simulink model "controller_observer_AllAgents_v5"  (Simulink 8.4)
settings: Solver=ode1  StartTime=0.0  StopTime=inf  FixedStep=60*1  RelTol=1e-3
values from neighbouring files (symbols this model uses):
  Cpw = 4180    [readepJSONout.m]
  m_max = 3    [DesignParameters.m]
  mc_MAX = 2    [DesignParameters.m]
  Tamb_additive_Sensor_fault_time = 60*60*24*(Tamb_day_ofthe_add_fault + Tamb_time_ofthe_add_fault)    (= 129600)    [faults_setting.m]
  Tamb_additive_Sensor_fault_value = 10    [faults_setting.m]
  Tamb_mult_Sensor_fault_time = 60*60*24*(Tamb_day_ofthe_mult_fault + Tamb_time_ofthe_mult_fault)    (= 129600)    [faults_setting.m]
  Tamb_mult_Sensor_fault_value = 1.1    [faults_setting.m]
  Ts = 3600/dat.Timestep.Timestep_0x20_1.number_of_timesteps_per_hour    [readepJSONout.m]
  Tst_C_additive_Sensor_fault_time = 60*60*24*(Tst_C_day_ofthe_add_fault + Tst_C_time_ofthe_add_fault)    (= 129600)    [faults_setting.m]
  Tst_C_additive_Sensor_fault_value = 10    [faults_setting.m]
  Tst_C_mult_Sensor_fault_time = 60*60*24*(Tst_C_day_ofthe_mult_fault + Tst_C_time_ofthe_mult_fault)    (= 129600)    [faults_setting.m]
  Tst_C_mult_Sensor_fault_value = 1.1    [faults_setting.m]
  Tst_H_additive_Sensor_fault_time = 60*60*24*(Tst_H_day_ofthe_add_fault + Tst_H_time_ofthe_add_fault)    (= 129600)    [faults_setting.m]
  Tst_H_additive_Sensor_fault_value = 10    [faults_setting.m]
  Tst_H_mult_Sensor_fault_time = 60*60*24*(Tst_H_day_ofthe_mult_fault + Tst_H_time_ofthe_mult_fault)    (= 129600)    [faults_setting.m]
  Tst_H_mult_Sensor_fault_value = 1.1    [faults_setting.m]
undefined symbols (not defined in the file or in its folder): building, COP, Ehp_max, fsa_E, fsa_ET, fsa_N, fsa_NT, fsa_W, fsa_WT, h_E, h_ET, h_N, h_NT, h_W, h_WT, K_NtoNT, K_NTtoN, Ke_E, Ke_ET, Ke_N, Ke_NT, Ke_WT, Km_E, Km_ET, Km_N, Km_NT, Km_W, Km_WT, Kout_N, Kout_NT, Kout_W, Kout_WT, Kst, Ksta, Kstcij, KstRef, Kw_E, Kw_ET, Kw_N, Kw_NT, Kw_W, Kw_WT
libraries: simulink x61
(part 28 of 36)

== system controller_observer_AllAgents_v5/Controller/CorridorPod1_Agent/Threshold_zone ==
  3 : Constant  SampleTime=Ts
  4 : Constant  Value=[ones(numel(building.zone(10).neighbour),1)]  SampleTime=Ts
  Add2 : Sum  Inputs=+-
  Add3 : Sum  Inputs=+-
  Divide : Product  Inputs=*/
  Dot Product1 : DotProduct  SampleTime=Ts  InputSameDT=on  OutDataTypeStr=Inherit: Inherit via internal rule
  Fcn2 : Fcn  Expr=u(2)^(u(1))  SampleTime=Ts
  Gain : Gain  Gain=building.zone(10).e_bar  SampleTime=Ts
  If Action Subsystem2 : Subsystem  TreatAsAtomicUnit=on
  If Action Subsystem3 : Subsystem  TreatAsAtomicUnit=on
  If1 : If  IfExpression=u1 == 0  SampleTime=Ts
  Merge : Merge  InitialOutput=[building.zone(4).e_bar+building.zone(4).n_bar]
  Mux2 : Mux  Inputs=2
  Mux3 : Mux  Inputs=[1 numel(building.zone(10).neighbour) 1 1]
  Product1 : Product  SampleTime=Ts
  Product5 : Product  SampleTime=Ts
  Sum3 : Sum  Inputs=|++  SampleTime=Ts
  Sum4 : Sum  Inputs=+++  SampleTime=Ts
  ZoneGainsThreshold1 : Constant  Value=[building.zone(10).B_z; transpose(building.zone(10).B_z*building.zone(10).n_nei.*building.zone(10).Kij); building.zone(10).B_z*building.zone(10).L; building.zone(10).B_z*building.zone(10).Qbar]  SampleTime=Ts  FramePeriod=Ts
  a mat : ToFile  Filename=C:\Users\papad\Dropbox\Complete_building_simulator_Observers\Results_fz4_sensor\aH_CorridorPod1.mat  SaveFormat=Timeseries  SampleTime=Ts
  a1 : Constant  Value=building.zone(10).a  SampleTime=Ts
  ae1 : Constant  Value=building.zone(10).a*building.zone(10).e_bar  SampleTime=Ts
  ae_sum1 : ToFile  Filename=C:\Users\papad\Dropbox\Complete_building_simulator_Observers\Results_fz4_sensor\aeH_sum_CorridorPod1.mat  SaveFormat=Timeseries  SampleTime=Ts
  ae_z2 : ToFile  Filename=C:\Users\papad\Dropbox\Complete_building_simulator_Observers\Results_fz4_sensor\aeH_CorridorPod1.mat  SaveFormat=Timeseries  SampleTime=Ts
  ae_z3 : ToFile  Filename=C:\Users\papad\Dropbox\Complete_building_simulator_Observers\Results_fz4_sensor\kH_CorridorPod1.mat  SaveFormat=Timeseries  SampleTime=Ts
  counter : Subsystem
  n_bar1 : Constant  Value=building.zone(10).n_bar  SampleTime=Ts
  n_bar2 : Constant  Value=building.zone(4).n_bar  SampleTime=Ts
  x1 : ToFile  Filename=C:\Users\papad\Dropbox\Complete_building_simulator_Observers\Results_fz4_sensor\xH_CorridorPod1.mat  SaveFormat=Timeseries  SampleTime=Ts
  Out1 : Outport
  wires:
  Mux3/1 -> Dot Product1/1
  ZoneGainsThreshold1/1 -> Dot Product1/2
  n_bar1/1 -> Mux3/1
  4/1 -> Mux3/2
  3/1 -> Mux3/4
  If1/1 -> If Action Subsystem2/Action
  If1/2 -> If Action Subsystem3/Action
  If Action Subsystem2/1(Out1) -> Merge/1  "k=0"
  If Action Subsystem3/1(Out1) -> Merge/2  "k>0"
  Sum3/1 -> If Action Subsystem2/1(In1)
  Sum4/1 -> If Action Subsystem3/1(In1)
  Gain/1 -> Sum4/1, ae_z2/1
  Fcn2/1 -> Add2/2, Gain/1  "a^k"
  Dot Product1/1 -> Product5/2, x1/1  "x"
  Product5/1 -> Sum4/3
  Merge/1 -> Out1/1
  a1/1 -> Mux2/2, Add2/1, Add3/1, Product1/2, Product1/1, a mat/1  "a"
  Mux2/1 -> Fcn2/1
  n_bar2/1 -> Sum4/2, Sum3/2, Mux3/3  "noise"
  Product1/1 -> Add3/2  "a^2"
  Add2/1 -> Divide/1
  Add3/1 -> Divide/2
  Divide/1 -> ae_sum1/1, Product5/1  "sum"
  ae1/1 -> Sum3/1
  counter/1(Out1) -> ae_z3/1, If1/1  "k"
  counter/2(Out2) -> Mux2/1  "k>1"

== system controller_observer_AllAgents_v5/Controller/CorridorPod1_Cooling/If Action Subsystem ==
  In1 : Inport
  Action Port : ActionPort  ActionType=then
  Out1 : Outport
  wires:
  In1/1 -> Out1/1

== system controller_observer_AllAgents_v5/Controller/CorridorPod1_Cooling/If Action Subsystem1 ==
  In1 : Inport
  Action Port : ActionPort  ActionType=else
  Out1 : Outport
  wires:
  In1/1 -> Out1/1

== system controller_observer_AllAgents_v5/Controller/CorridorPod1_Heating/If Action Subsystem ==
  In1 : Inport
  Action Port : ActionPort  ActionType=then
  Out1 : Outport
  wires:
  In1/1 -> Out1/1

== system controller_observer_AllAgents_v5/Controller/CorridorPod1_Heating/If Action Subsystem1 ==
  In1 : Inport
  Action Port : ActionPort  ActionType=else
  Out1 : Outport
  wires:
  In1/1 -> Out1/1

== system controller_observer_AllAgents_v5/Controller/CorridorPod2_Agent/Enabled Subsystem ==
  In1 : Inport
  Enable : EnablePort
  Out1 : Outport
  wires:
  In1/1 -> Out1/1

== system controller_observer_AllAgents_v5/Controller/CorridorPod2_Agent/Enabled Subsystem1 ==
  In1 : Inport
  Enable : EnablePort
  Out1 : Outport
  wires:
  In1/1 -> Out1/1

== system controller_observer_AllAgents_v5/Controller/CorridorPod2_Agent/Enabled Subsystem2 ==
  In1 : Inport
  Enable : EnablePort
  Out1 : Outport
  wires:
  In1/1 -> Out1/1

== system controller_observer_AllAgents_v5/Controller/CorridorPod2_Agent/Enabled Subsystem3 ==
  In1 : Inport
  Enable : EnablePort
  Out1 : Outport
  wires:
  In1/1 -> Out1/1

== system controller_observer_AllAgents_v5/Controller/CorridorPod2_Agent/If Action Subsystem1 ==
  In1 : Inport
  Action Port : ActionPort
  Out1 : Outport
  wires:
  In1/1 -> Out1/1

== system controller_observer_AllAgents_v5/Controller/CorridorPod2_Agent/If Action Subsystem2 ==
  In1 : Inport
  Action Port : ActionPort
  Out1 : Outport
  wires:
  In1/1 -> Out1/1

== system controller_observer_AllAgents_v5/Controller/CorridorPod2_Agent/If Action Subsystem3 ==
  In1 : Inport
  Action Port : ActionPort
  Out1 : Outport
  wires:
  In1/1 -> Out1/1

== system controller_observer_AllAgents_v5/Controller/CorridorPod2_Agent/If Action Subsystem4 ==
  In1 : Inport
  Action Port : ActionPort
  Out1 : Outport
  wires:
  In1/1 -> Out1/1

== system controller_observer_AllAgents_v5/Controller/CorridorPod2_Agent/If Action Subsystem5 ==
  In1 : Inport
  Action Port : ActionPort
  Out1 : Outport
  wires:
  In1/1 -> Out1/1

== system controller_observer_AllAgents_v5/Controller/CorridorPod2_Agent/If Action Subsystem6 ==
  In1 : Inport
  Action Port : ActionPort
  Out1 : Outport
  wires:
  In1/1 -> Out1/1

== system controller_observer_AllAgents_v5/Controller/CorridorPod2_Agent/If Action Subsystem7 ==
  In1 : Inport
  Action Port : ActionPort
  Out1 : Outport
  wires:
  In1/1 -> Out1/1

== system controller_observer_AllAgents_v5/Controller/CorridorPod2_Agent/If Action Subsystem8 ==
  In1 : Inport
  Action Port : ActionPort
  Out1 : Outport
  wires:
  In1/1 -> Out1/1

== system controller_observer_AllAgents_v5/Controller/CorridorPod2_Agent/Threshold_coil ==
  mc : Inport
  Add2 : Sum  Inputs=+-
  Add3 : Sum  Inputs=+-
  CoilGainsThreshold : Constant  Value=[norm(building.zone(11).B_c_C); norm(building.zone(11).B_c_C*building.zone(11).Dma_C); norm(building.zone(11).L_c_C)]  SampleTime=Ts  FramePeriod=Ts
  Divide : Product  Inputs=*/
  Dot Product1 : DotProduct  SampleTime=Ts  InputSameDT=on  OutDataTypeStr=Inherit: Inherit via internal rule
  Fcn2 : Fcn  Expr=u(2)^(u(1))  SampleTime=Ts
  Gain : Gain  Gain=building.zone(11).e_c_C_bar  SampleTime=Ts
  If Action Subsystem2 : Subsystem  TreatAsAtomicUnit=on
  If Action Subsystem3 : Subsystem  TreatAsAtomicUnit=on
  If1 : If  IfExpression=u1 == 0  SampleTime=Ts
  Merge : Merge  InitialOutput=[building.zone(4).e_bar+building.zone(4).n_bar]
  Mux2 : Mux  Inputs=2
  Mux3 : Mux  Inputs=[1 1 1]
  Product1 : Product  SampleTime=Ts
  Product2 : Product  SampleTime=Ts
  Product5 : Product  SampleTime=Ts
  Sum3 : Sum  Inputs=|++  SampleTime=Ts
  Sum4 : Sum  Inputs=+++  SampleTime=Ts
  a_c_C : Constant  Value=building.zone(11).a_c_C  SampleTime=Ts
  ae_C_C : ToFile  Filename=C:\Users\papad\Dropbox\Complete_building_simulator_Observers\Results_fz4_sensor\ae_C_C_CorridorPod2.mat  SaveFormat=Timeseries  SampleTime=Ts
  ae_C_C_sum : ToFile  Filename=C:\Users\papad\Dropbox\Complete_building_simulator_Observers\Results_fz4_sensor\ae_C_C_sum_CorridorPod2.mat  SaveFormat=Timeseries  SampleTime=Ts
  ae_c_C : Constant  Value=building.zone(11).e_c_C_bar  SampleTime=Ts
  counter : Subsystem
  k_c_C : ToFile  Filename=C:\Users\papad\Dropbox\Complete_building_simulator_Observers\Results_fz4_sensor\k_c_C_CorridorPod2.mat  SaveFormat=Timeseries  SampleTime=Ts
  nC_bar : Constant  Value=building.zone(11).nC_bar  SampleTime=Ts
  nC_bar1 : Constant  Value=building.zone(4).n_bar  SampleTime=Ts
  nZ_bar : Constant  Value=building.zone(11).n_bar  SampleTime=Ts
  x_C_C : ToFile  Filename=C:\Users\papad\Dropbox\Complete_building_simulator_Observers\Results_fz4_sensor\x_C_C_CorridorPod2.mat  SaveFormat=Timeseries  SampleTime=Ts
  Out1 : Outport
  wires:
  Mux3/1 -> Dot Product1/1
  CoilGainsThreshold/1 -> Dot Product1/2
  nZ_bar/1 -> Mux3/2
  If1/1 -> If Action Subsystem2/Action
  If1/2 -> If Action Subsystem3/Action
  If Action Subsystem2/1(Out1) -> Merge/1  "k=0"
  If Action Subsystem3/1(Out1) -> Merge/2  "k>0"
  Sum3/1 -> If Action Subsystem2/1(In1)
  Sum4/1 -> If Action Subsystem3/1(In1)
  Gain/1 -> Sum4/1, ae_C_C/1
  Fcn2/1 -> Add2/2, Gain/1  "a^k"
  Dot Product1/1 -> Product5/2, x_C_C/1  "x"
  Product5/1 -> Sum4/3
  Merge/1 -> Out1/1
  a_c_C/1 -> Mux2/2, Add2/1, Add3/1, Product1/2, Product1/1  "a"
  Mux2/1 -> Fcn2/1
  nC_bar/1 -> Sum4/2, Sum3/2, Mux3/3  "noise"
  Product1/1 -> Add3/2  "a^2"
  Add2/1 -> Divide/1
  Add3/1 -> Divide/2
  Divide/1 -> ae_C_C_sum/1, Product5/1  "sum"
  ae_c_C/1 -> Sum3/1
  counter/1(Out1) -> k_c_C/1, If1/1  "k"
  counter/2(Out2) -> Mux2/1  "k>1"
  Product2/1 -> Mux3/1
  nC_bar1/1 -> Product2/1
  mc/1 -> Product2/2

== system controller_observer_AllAgents_v5/Controller/CorridorPod2_Agent/Threshold_hcoil ==
  mhc : Inport
  mcc : Inport  Port=2
  Add2 : Sum  Inputs=+-
  Add3 : Sum  Inputs=+-
  CoilGainsThreshold : Constant  Value=[norm(building.zone(11).B_c_H); norm(building.zone(11).B_c_H); norm(building.zone(11).B_c_H*building.zone(11).Dmac_H); norm(building.zone(11).L_c_H)]  SampleTime=Ts  FramePeriod=Ts
  Divide : Product  Inputs=*/
  Dot Product1 : DotProduct  SampleTime=Ts  InputSameDT=on  OutDataTypeStr=Inherit: Inherit via internal rule
  Fcn2 : Fcn  Expr=u(2)^(u(1))  SampleTime=Ts
  Gain : Gain  Gain=building.zone(11).e_c_H_bar  SampleTime=Ts
  If Action Subsystem2 : Subsystem  TreatAsAtomicUnit=on
  If Action Subsystem3 : Subsystem  TreatAsAtomicUnit=on
  If1 : If  IfExpression=u1 == 0  SampleTime=Ts
  Merge : Merge  InitialOutput=[building.zone(4).e_bar+building.zone(4).n_bar]
  Mux2 : Mux  Inputs=2
  Mux3 : Mux  Inputs=[1 1 1 1]
  Product{1..3,5} (4 alike) : Product  SampleTime=Ts
  Sum3 : Sum  Inputs=|++  SampleTime=Ts
  Sum4 : Sum  Inputs=+++  SampleTime=Ts
  a_c_C : Constant  Value=building.zone(11).a_c_H  SampleTime=Ts
  ae_C_C : ToFile  Filename=C:\Users\papad\Dropbox\Complete_building_simulator_Observers\Results_fz4_sensor\ae_C_H_CorridorPod2.mat  SaveFormat=Timeseries  SampleTime=Ts
  ae_C_C_sum : ToFile  Filename=C:\Users\papad\Dropbox\Complete_building_simulator_Observers\Results_fz4_sensor\ae_C_H_sum_CorridorPod2.mat  SaveFormat=Timeseries  SampleTime=Ts
  ae_c_C : Constant  Value=building.zone(11).e_c_H_bar  SampleTime=Ts
  counter : Subsystem
  k_c_C : ToFile  Filename=C:\Users\papad\Dropbox\Complete_building_simulator_Observers\Results_fz4_sensor\k_c_H_CorridorPod2.mat  SaveFormat=Timeseries  SampleTime=Ts
  nC_bar : Constant  Value=building.zone(11).nH_bar  SampleTime=Ts
  nC_bar1 : Constant  Value=building.zone(4).n_bar  SampleTime=Ts
  nC_bar2 : Constant  Value=building.zone(4).n_bar  SampleTime=Ts
  nZ_bar : Constant  Value=building.zone(11).n_bar  SampleTime=Ts
  x_C_C : ToFile  Filename=C:\Users\papad\Dropbox\Complete_building_simulator_Observers\Results_fz4_sensor\x_C_H_CorridorPod2.mat  SaveFormat=Timeseries  SampleTime=Ts
  Out1 : Outport
  wires:
  Mux3/1 -> Dot Product1/1
  CoilGainsThreshold/1 -> Dot Product1/2
  nZ_bar/1 -> Mux3/3
  If1/1 -> If Action Subsystem2/Action
  If1/2 -> If Action Subsystem3/Action
  If Action Subsystem2/1(Out1) -> Merge/1  "k=0"
  If Action Subsystem3/1(Out1) -> Merge/2  "k>0"
  Sum3/1 -> If Action Subsystem2/1(In1)
  Sum4/1 -> If Action Subsystem3/1(In1)
  Gain/1 -> Sum4/1, ae_C_C/1
  Fcn2/1 -> Add2/2, Gain/1  "a^k"
  Dot Product1/1 -> Product5/2, x_C_C/1  "x"
  Product5/1 -> Sum4/3
  Merge/1 -> Out1/1
  a_c_C/1 -> Mux2/2, Add2/1, Add3/1, Product1/2, Product1/1  "a"
  Mux2/1 -> Fcn2/1
  nC_bar/1 -> Mux3/4, Sum4/2, Sum3/2  "noise"
  Product1/1 -> Add3/2  "a^2"
  Add2/1 -> Divide/1
  Add3/1 -> Divide/2
  Divide/1 -> ae_C_C_sum/1, Product5/1  "sum"
  ae_c_C/1 -> Sum3/1
  counter/1(Out1) -> k_c_C/1, If1/1  "k"
  counter/2(Out2) -> Mux2/1  "k>1"
  Product2/1 -> Mux3/1
  nC_bar1/1 -> Product2/1
  mhc/1 -> Product2/2
  nC_bar2/1 -> Product3/1
  mcc/1 -> Product3/2
  Product3/1 -> Mux3/2

== system controller_observer_AllAgents_v5/Controller/CorridorPod2_Agent/Threshold_sa ==
  Add2 : Sum  Inputs=+-
  Add3 : Sum  Inputs=+-
  Divide : Product  Inputs=*/
  Dot Product1 : DotProduct  SampleTime=Ts  InputSameDT=on  OutDataTypeStr=Inherit: Inherit via internal rule
  Fcn2 : Fcn  Expr=u(2)^(u(1))  SampleTime=Ts
  Gain : Gain  Gain=building.zone(11).e_SA_C_bar  SampleTime=Ts
  If Action Subsystem2 : Subsystem  TreatAsAtomicUnit=on
  If Action Subsystem3 : Subsystem  TreatAsAtomicUnit=on
  If1 : If  IfExpression=u1 == 0  SampleTime=Ts
  Merge : Merge  InitialOutput=[building.zone(4).e_bar+building.zone(4).n_bar]
  Mux2 : Mux  Inputs=2
  Mux3 : Mux  Inputs=[ 1 1 1 1]
  Product1 : Product  SampleTime=Ts
  Product5 : Product  SampleTime=Ts
  SAGainsThreshold : Constant  Value=[norm(building.zone(11).B_sa_C); norm(building.zone(11).B_sa_C); norm(building.zone(11).B_sa_C*building.zone(11).Dma_SA); norm(building.zone(11).L_sa_C)]  SampleTime=Ts  FramePeriod=Ts
  Sum3 : Sum  Inputs=|++  SampleTime=Ts
  Sum4 : Sum  Inputs=+++  SampleTime=Ts
  a_SA_C : Constant  Value=building.zone(11).a_SA_C  SampleTime=Ts
  aeSA_C_sum : ToFile  Filename=C:\Users\papad\Dropbox\Complete_building_simulator_Observers\Results_fz4_sensor\aeSA_C_sum_CorridorPod2.mat  SaveFormat=Timeseries  SampleTime=Ts
  ae_SAC : Constant  Value=building.zone(11).e_SA_C_bar  SampleTime=Ts
  ae_SA_C : ToFile  Filename=C:\Users\papad\Dropbox\Complete_building_simulator_Observers\Results_fz4_sensor\ae_SA_C_CorridorPod2.mat  SaveFormat=Timeseries  SampleTime=Ts
  counter : Subsystem
  k_SA_C : ToFile  Filename=C:\Users\papad\Dropbox\Complete_building_simulator_Observers\Results_fz4_sensor\k_SA_C_CorridorPod2.mat  SaveFormat=Timeseries  SampleTime=Ts
  nC_bar : Constant  Value=building.zone(11).nC_bar+0.5  SampleTime=Ts
  nH_bar : Constant  Value=building.zone(4).nH_bar  SampleTime=Ts
  nSA_bar : Constant  Value=building.zone(11).nC_bar  SampleTime=Ts
  nZ_bar : Constant  Value=building.zone(11).n_bar  SampleTime=Ts
  xSA_C : ToFile  Filename=C:\Users\papad\Dropbox\Complete_building_simulator_Observers\Results_fz4_sensor\xSA_C_CorridorPod2.mat  SaveFormat=Timeseries  SampleTime=Ts
  Out1 : Outport
  wires:
  Mux3/1 -> Dot Product1/1
  SAGainsThreshold/1 -> Dot Product1/2
  nC_bar/1 -> Mux3/1
  If1/1 -> If Action Subsystem2/Action
  If1/2 -> If Action Subsystem3/Action
  If Action Subsystem2/1(Out1) -> Merge/1  "k=0"
  If Action Subsystem3/1(Out1) -> Merge/2  "k>0"
  Sum3/1 -> If Action Subsystem2/1(In1)
  Sum4/1 -> If Action Subsystem3/1(In1)
  Gain/1 -> Sum4/1, ae_SA_C/1
  Fcn2/1 -> Add2/2, Gain/1  "a^k"
  Dot Product1/1 -> Product5/2, xSA_C/1  "x"
  Product5/1 -> Sum4/3
  Merge/1 -> Out1/1
  a_SA_C/1 -> Mux2/2, Add2/1, Add3/1, Product1/2, Product1/1  "a"
  Mux2/1 -> Fcn2/1
  nSA_bar/1 -> Mux3/4, Sum4/2, Sum3/2  "noise"
  Product1/1 -> Add3/2  "a^2"
  Add2/1 -> Divide/1
  Add3/1 -> Divide/2
  Divide/1 -> aeSA_C_sum/1, Product5/1  "sum"
  ae_SAC/1 -> Sum3/1
  counter/1(Out1) -> k_SA_C/1, If1/1  "k"
  counter/2(Out2) -> Mux2/1  "k>1"
  nH_bar/1 -> Mux3/2
  nZ_bar/1 -> Mux3/3

== system controller_observer_AllAgents_v5/Controller/CorridorPod2_Agent/Threshold_zone ==
  3 : Constant  SampleTime=Ts
  4 : Constant  Value=[ones(numel(building.zone(11).neighbour),1)]  SampleTime=Ts
  Add2 : Sum  Inputs=+-
  Add3 : Sum  Inputs=+-
  Divide : Product  Inputs=*/
  Dot Product1 : DotProduct  SampleTime=Ts  InputSameDT=on  OutDataTypeStr=Inherit: Inherit via internal rule
  Fcn2 : Fcn  Expr=u(2)^(u(1))  SampleTime=Ts
  Gain : Gain  Gain=building.zone(11).e_bar  SampleTime=Ts
  If Action Subsystem2 : Subsystem  TreatAsAtomicUnit=on
  If Action Subsystem3 : Subsystem  TreatAsAtomicUnit=on
  If1 : If  IfExpression=u1 == 0  SampleTime=Ts
  Merge : Merge  InitialOutput=[building.zone(4).e_bar+building.zone(4).n_bar]
  Mux2 : Mux  Inputs=2
  Mux3 : Mux  Inputs=[1 numel(building.zone(11).neighbour) 1 1]
  Product1 : Product  SampleTime=Ts
  Product5 : Product  SampleTime=Ts
  Sum3 : Sum  Inputs=|++  SampleTime=Ts
  Sum4 : Sum  Inputs=+++  SampleTime=Ts
  ZoneGainsThreshold1 : Constant  Value=[building.zone(11).B_z; transpose(building.zone(11).B_z*building.zone(11).n_nei.*building.zone(11).Kij); building.zone(11).B_z*building.zone(11).L; building.zone(11).B_z*building.zone(11).Qbar]  SampleTime=Ts  FramePeriod=Ts
  a mat : ToFile  Filename=C:\Users\papad\Dropbox\Complete_building_simulator_Observers\Results_fz4_sensor\aH_CorridorPod2.mat  SaveFormat=Timeseries  SampleTime=Ts
  a1 : Constant  Value=building.zone(11).a  SampleTime=Ts
  ae1 : Constant  Value=building.zone(11).a*building.zone(11).e_bar  SampleTime=Ts
  ae_sum1 : ToFile  Filename=C:\Users\papad\Dropbox\Complete_building_simulator_Observers\Results_fz4_sensor\aeH_sum_CorridorPod2.mat  SaveFormat=Timeseries  SampleTime=Ts
  ae_z2 : ToFile  Filename=C:\Users\papad\Dropbox\Complete_building_simulator_Observers\Results_fz4_sensor\aeH_CorridorPod2.mat  SaveFormat=Timeseries  SampleTime=Ts
  ae_z3 : ToFile  Filename=C:\Users\papad\Dropbox\Complete_building_simulator_Observers\Results_fz4_sensor\kH_CorridorPod2.mat  SaveFormat=Timeseries  SampleTime=Ts
  counter : Subsystem
  n_bar1 : Constant  Value=building.zone(11).n_bar  SampleTime=Ts
  n_bar2 : Constant  Value=building.zone(4).n_bar  SampleTime=Ts
  x1 : ToFile  Filename=C:\Users\papad\Dropbox\Complete_building_simulator_Observers\Results_fz4_sensor\xH_CorridorPod2.mat  SaveFormat=Timeseries  SampleTime=Ts
  Out1 : Outport
  wires:
  Mux3/1 -> Dot Product1/1
  ZoneGainsThreshold1/1 -> Dot Product1/2
  n_bar1/1 -> Mux3/1
  4/1 -> Mux3/2
  3/1 -> Mux3/4
  If1/1 -> If Action Subsystem2/Action
  If1/2 -> If Action Subsystem3/Action
  If Action Subsystem2/1(Out1) -> Merge/1  "k=0"
  If Action Subsystem3/1(Out1) -> Merge/2  "k>0"
  Sum3/1 -> If Action Subsystem2/1(In1)
  Sum4/1 -> If Action Subsystem3/1(In1)
  Gain/1 -> Sum4/1, ae_z2/1
  Fcn2/1 -> Add2/2, Gain/1  "a^k"
  Dot Product1/1 -> Product5/2, x1/1  "x"
  Product5/1 -> Sum4/3
  Merge/1 -> Out1/1
  a1/1 -> Mux2/2, Add2/1, Add3/1, Product1/2, Product1/1, a mat/1  "a"
  Mux2/1 -> Fcn2/1
  n_bar2/1 -> Sum4/2, Sum3/2, Mux3/3  "noise"
  Product1/1 -> Add3/2  "a^2"
  Add2/1 -> Divide/1
  Add3/1 -> Divide/2
  Divide/1 -> ae_sum1/1, Product5/1  "sum"
  ae1/1 -> Sum3/1
  counter/1(Out1) -> ae_z3/1, If1/1  "k"
  counter/2(Out2) -> Mux2/1  "k>1"

== system controller_observer_AllAgents_v5/Controller/CorridorPod2_Cooling/If Action Subsystem ==
  In1 : Inport
  Action Port : ActionPort  ActionType=then
  Out1 : Outport
  wires:
  In1/1 -> Out1/1

== system controller_observer_AllAgents_v5/Controller/CorridorPod2_Cooling/If Action Subsystem1 ==
  In1 : Inport
  Action Port : ActionPort  ActionType=else
  Out1 : Outport
  wires:
  In1/1 -> Out1/1

== system controller_observer_AllAgents_v5/Controller/CorridorPod2_Heating/If Action Subsystem ==
  In1 : Inport
  Action Port : ActionPort  ActionType=then
  Out1 : Outport
  wires:
  In1/1 -> Out1/1

== system controller_observer_AllAgents_v5/Controller/CorridorPod2_Heating/If Action Subsystem1 ==
  In1 : Inport
  Action Port : ActionPort  ActionType=else
  Out1 : Outport
  wires:
  In1/1 -> Out1/1

== system controller_observer_AllAgents_v5/Controller/CorridorPod3_Agent/Enabled Subsystem ==
  In1 : Inport
  Enable : EnablePort
  Out1 : Outport
  wires:
  In1/1 -> Out1/1

== system controller_observer_AllAgents_v5/Controller/CorridorPod3_Agent/Enabled Subsystem1 ==
  In1 : Inport
  Enable : EnablePort
  Out1 : Outport
  wires:
  In1/1 -> Out1/1

== system controller_observer_AllAgents_v5/Controller/CorridorPod3_Agent/Enabled Subsystem2 ==
  In1 : Inport
  Enable : EnablePort
  Out1 : Outport
  wires:
  In1/1 -> Out1/1

== system controller_observer_AllAgents_v5/Controller/CorridorPod3_Agent/Enabled Subsystem3 ==
  In1 : Inport
  Enable : EnablePort
  Out1 : Outport
  wires:
  In1/1 -> Out1/1

== system controller_observer_AllAgents_v5/Controller/CorridorPod3_Agent/If Action Subsystem1 ==
  In1 : Inport
  Action Port : ActionPort
  Out1 : Outport
  wires:
  In1/1 -> Out1/1

== system controller_observer_AllAgents_v5/Controller/CorridorPod3_Agent/If Action Subsystem2 ==
  In1 : Inport
  Action Port : ActionPort
  Out1 : Outport
  wires:
  In1/1 -> Out1/1

== system controller_observer_AllAgents_v5/Controller/CorridorPod3_Agent/If Action Subsystem3 ==
  In1 : Inport
  Action Port : ActionPort
  Out1 : Outport
  wires:
  In1/1 -> Out1/1

== system controller_observer_AllAgents_v5/Controller/CorridorPod3_Agent/If Action Subsystem4 ==
  In1 : Inport
  Action Port : ActionPort
  Out1 : Outport
  wires:
  In1/1 -> Out1/1

== system controller_observer_AllAgents_v5/Controller/CorridorPod3_Agent/If Action Subsystem5 ==
  In1 : Inport
  Action Port : ActionPort
  Out1 : Outport
  wires:
  In1/1 -> Out1/1

== system controller_observer_AllAgents_v5/Controller/CorridorPod3_Agent/If Action Subsystem6 ==
  In1 : Inport
  Action Port : ActionPort
  Out1 : Outport
  wires:
  In1/1 -> Out1/1

== system controller_observer_AllAgents_v5/Controller/CorridorPod3_Agent/If Action Subsystem7 ==
  In1 : Inport
  Action Port : ActionPort
  Out1 : Outport
  wires:
  In1/1 -> Out1/1

== system controller_observer_AllAgents_v5/Controller/CorridorPod3_Agent/If Action Subsystem8 ==
  In1 : Inport
  Action Port : ActionPort
  Out1 : Outport
  wires:
  In1/1 -> Out1/1

== system controller_observer_AllAgents_v5/Controller/CorridorPod3_Agent/Threshold_coil ==
  mc : Inport
  Abs : Abs
  Abs1 : Abs
  Add2 : Sum  Inputs=+-
  Add3 : Sum  Inputs=+-
  CoilGainsThreshold : Constant  Value=[norm(building.zone(12).B_c_C); norm(building.zone(12).B_c_C*building.zone(12).Dma_C); norm(building.zone(12).L_c_C)]  SampleTime=Ts  FramePeriod=Ts
  Divide : Product  Inputs=*/
  Dot Product1 : DotProduct  SampleTime=Ts  InputSameDT=on  OutDataTypeStr=Inherit: Inherit via internal rule
  Fcn2 : Fcn  Expr=u(2)^(u(1))  SampleTime=Ts
  Gain : Gain  Gain=building.zone(12).e_c_C_bar  SampleTime=Ts
  If Action Subsystem2 : Subsystem  TreatAsAtomicUnit=on
  If Action Subsystem3 : Subsystem  TreatAsAtomicUnit=on
  If1 : If  IfExpression=u1 == 0  SampleTime=Ts
  Merge : Merge  InitialOutput=[building.zone(4).e_bar+building.zone(4).n_bar]
  Mux2 : Mux  Inputs=2
  Mux3 : Mux  Inputs=[1 1 1]
  Product1 : Product  SampleTime=Ts
  Product2 : Product  SampleTime=Ts
  Product5 : Product  SampleTime=Ts
  Sum3 : Sum  Inputs=|++  SampleTime=Ts
  Sum4 : Sum  Inputs=+++  SampleTime=Ts
  a_c_C : Constant  Value=building.zone(12).a_c_C  SampleTime=Ts
  ae_C_C : ToFile  Filename=C:\Users\papad\Dropbox\Complete_building_simulator_Observers\Results_fz4_sensor\ae_C_C_CorridorPod3.mat  SaveFormat=Timeseries  SampleTime=Ts
  ae_C_C_sum : ToFile  Filename=C:\Users\papad\Dropbox\Complete_building_simulator_Observers\Results_fz4_sensor\ae_C_C_sum_CorridorPod3.mat  SaveFormat=Timeseries  SampleTime=Ts
  ae_c_C : Constant  Value=building.zone(12).e_c_C_bar  SampleTime=Ts
  counter : Subsystem
  k_c_C : ToFile  Filename=C:\Users\papad\Dropbox\Complete_building_simulator_Observers\Results_fz4_sensor\k_c_C_CorridorPod3.mat  SaveFormat=Timeseries  SampleTime=Ts
  nC_bar : Constant  Value=building.zone(12).nC_bar  SampleTime=Ts
  nC_bar1 : Constant  Value=building.zone(4).n_bar  SampleTime=Ts
  nZ_bar : Constant  Value=building.zone(12).n_bar  SampleTime=Ts
  x_C_C : ToFile  Filename=C:\Users\papad\Dropbox\Complete_building_simulator_Observers\Results_fz4_sensor\x_C_C_CorridorPod3.mat  SaveFormat=Timeseries  SampleTime=Ts
  Out1 : Outport
  wires:
  Mux3/1 -> Dot Product1/1
  CoilGainsThreshold/1 -> Dot Product1/2
  nZ_bar/1 -> Mux3/2
  If1/1 -> If Action Subsystem2/Action
  If1/2 -> If Action Subsystem3/Action
  If Action Subsystem2/1(Out1) -> Merge/1  "k=0"
  If Action Subsystem3/1(Out1) -> Merge/2  "k>0"
  Sum3/1 -> If Action Subsystem2/1(In1)
  Sum4/1 -> If Action Subsystem3/1(In1)
  Gain/1 -> Sum4/1, ae_C_C/1
  Fcn2/1 -> Add2/2, Gain/1  "a^k"
  Dot Product1/1 -> Product5/2, x_C_C/1  "x"
  Product5/1 -> Sum4/3
  Merge/1 -> Out1/1
  a_c_C/1 -> Mux2/2, Add2/1, Add3/1, Product1/2, Product1/1  "a"
  Mux2/1 -> Fcn2/1
  nC_bar/1 -> Sum4/2, Sum3/2, Mux3/3  "noise"
  Product1/1 -> Add3/2  "a^2"
  Add2/1 -> Divide/1
  Add3/1 -> Divide/2
  Divide/1 -> ae_C_C_sum/1, Product5/1  "sum"
  ae_c_C/1 -> Sum3/1
  counter/1(Out1) -> k_c_C/1, If1/1  "k"
  counter/2(Out2) -> Mux2/1  "k>1"
  Product2/1 -> Mux3/1
  nC_bar1/1 -> Product2/1
  mc/1 -> Abs1/1
  Abs1/1 -> Product2/2

== system controller_observer_AllAgents_v5/Controller/CorridorPod3_Agent/Threshold_hcoil ==
  mhc : Inport
  mcc : Inport  Port=2
  Add2 : Sum  Inputs=+-
  Add3 : Sum  Inputs=+-
  CoilGainsThreshold : Constant  Value=[norm(building.zone(12).B_c_H); norm(building.zone(12).B_c_H); norm(building.zone(12).B_c_H*building.zone(12).Dmac_H); norm(building.zone(12).L_c_H)]  SampleTime=Ts  FramePeriod=Ts
  Divide : Product  Inputs=*/
  Dot Product1 : DotProduct  SampleTime=Ts  InputSameDT=on  OutDataTypeStr=Inherit: Inherit via internal rule
  Fcn2 : Fcn  Expr=u(2)^(u(1))  SampleTime=Ts
  Gain : Gain  Gain=building.zone(12).e_c_H_bar  SampleTime=Ts
  If Action Subsystem2 : Subsystem  TreatAsAtomicUnit=on
  If Action Subsystem3 : Subsystem  TreatAsAtomicUnit=on
  If1 : If  IfExpression=u1 == 0  SampleTime=Ts
  Merge : Merge  InitialOutput=[building.zone(4).e_bar+building.zone(4).n_bar]
  Mux2 : Mux  Inputs=2
  Mux3 : Mux  Inputs=[1 1 1 1]
  Product{1..3,5} (4 alike) : Product  SampleTime=Ts
  Sum3 : Sum  Inputs=|++  SampleTime=Ts
  Sum4 : Sum  Inputs=+++  SampleTime=Ts
  a_c_C : Constant  Value=building.zone(12).a_c_H  SampleTime=Ts
  ae_C_C : ToFile  Filename=C:\Users\papad\Dropbox\Complete_building_simulator_Observers\Results_fz4_sensor\ae_C_H_CorridorPod3.mat  SaveFormat=Timeseries  SampleTime=Ts
  ae_C_C_sum : ToFile  Filename=C:\Users\papad\Dropbox\Complete_building_simulator_Observers\Results_fz4_sensor\ae_C_H_sum_CorridorPod3.mat  SaveFormat=Timeseries  SampleTime=Ts
  ae_c_C : Constant  Value=building.zone(12).e_c_H_bar  SampleTime=Ts
  counter : Subsystem
  k_c_C : ToFile  Filename=C:\Users\papad\Dropbox\Complete_building_simulator_Observers\Results_fz4_sensor\k_c_H_CorridorPod3.mat  SaveFormat=Timeseries  SampleTime=Ts
  nC_bar : Constant  Value=building.zone(12).nH_bar  SampleTime=Ts
  nC_bar1 : Constant  Value=building.zone(4).n_bar  SampleTime=Ts
  nC_bar2 : Constant  Value=building.zone(4).n_bar  SampleTime=Ts
  nZ_bar : Constant  Value=building.zone(12).n_bar  SampleTime=Ts
  x_C_C : ToFile  Filename=C:\Users\papad\Dropbox\Complete_building_simulator_Observers\Results_fz4_sensor\x_C_H_CorridorPod3.mat  SaveFormat=Timeseries  SampleTime=Ts
  Out1 : Outport
  wires:
  Mux3/1 -> Dot Product1/1
  CoilGainsThreshold/1 -> Dot Product1/2
  nZ_bar/1 -> Mux3/3
  If1/1 -> If Action Subsystem2/Action
  If1/2 -> If Action Subsystem3/Action
  If Action Subsystem2/1(Out1) -> Merge/1  "k=0"
  If Action Subsystem3/1(Out1) -> Merge/2  "k>0"
  Sum3/1 -> If Action Subsystem2/1(In1)
  Sum4/1 -> If Action Subsystem3/1(In1)
  Gain/1 -> Sum4/1, ae_C_C/1
  Fcn2/1 -> Add2/2, Gain/1  "a^k"
  Dot Product1/1 -> Product5/2, x_C_C/1  "x"
  Product5/1 -> Sum4/3
  Merge/1 -> Out1/1
  a_c_C/1 -> Mux2/2, Add2/1, Add3/1, Product1/2, Product1/1  "a"
  Mux2/1 -> Fcn2/1
  nC_bar/1 -> Mux3/4, Sum4/2, Sum3/2  "noise"
  Product1/1 -> Add3/2  "a^2"
  Add2/1 -> Divide/1
  Add3/1 -> Divide/2
  Divide/1 -> ae_C_C_sum/1, Product5/1  "sum"
  ae_c_C/1 -> Sum3/1
  counter/1(Out1) -> k_c_C/1, If1/1  "k"
  counter/2(Out2) -> Mux2/1  "k>1"
  Product2/1 -> Mux3/1
  nC_bar1/1 -> Product2/1
  mhc/1 -> Product2/2
  nC_bar2/1 -> Product3/1
  mcc/1 -> Product3/2
  Product3/1 -> Mux3/2

== system controller_observer_AllAgents_v5/Controller/CorridorPod3_Agent/Threshold_sa ==
  Add2 : Sum  Inputs=+-
  Add3 : Sum  Inputs=+-
  Divide : Product  Inputs=*/
  Dot Product1 : DotProduct  SampleTime=Ts  InputSameDT=on  OutDataTypeStr=Inherit: Inherit via internal rule
  Fcn2 : Fcn  Expr=u(2)^(u(1))  SampleTime=Ts
  Gain : Gain  Gain=building.zone(12).e_SA_C_bar  SampleTime=Ts
  If Action Subsystem2 : Subsystem  TreatAsAtomicUnit=on
  If Action Subsystem3 : Subsystem  TreatAsAtomicUnit=on
  If1 : If  IfExpression=u1 == 0  SampleTime=Ts
  Merge : Merge  InitialOutput=[building.zone(4).e_bar+building.zone(4).n_bar]
  Mux2 : Mux  Inputs=2
  Mux3 : Mux  Inputs=[ 1 1 1 1]
  Product1 : Product  SampleTime=Ts
  Product5 : Product  SampleTime=Ts
  SAGainsThreshold : Constant  Value=[norm(building.zone(12).B_sa_C); norm(building.zone(12).B_sa_C); norm(building.zone(12).B_sa_C*building.zone(12).Dma_SA); norm(building.zone(12).L_sa_C)]  SampleTime=Ts  FramePeriod=Ts
  Sum3 : Sum  Inputs=|++  SampleTime=Ts
  Sum4 : Sum  Inputs=+++  SampleTime=Ts
  a_SA_C : Constant  Value=building.zone(12).a_SA_C  SampleTime=Ts
  aeSA_C_sum : ToFile  Filename=C:\Users\papad\Dropbox\Complete_building_simulator_Observers\Results_fz4_sensor\aeSA_C_sum_CorridorPod3.mat  SaveFormat=Timeseries  SampleTime=Ts
  ae_SAC : Constant  Value=building.zone(12).e_SA_C_bar  SampleTime=Ts
  ae_SA_C : ToFile  Filename=C:\Users\papad\Dropbox\Complete_building_simulator_Observers\Results_fz4_sensor\ae_SA_C_CorridorPod3.mat  SaveFormat=Timeseries  SampleTime=Ts
  counter : Subsystem
  k_SA_C : ToFile  Filename=C:\Users\papad\Dropbox\Complete_building_simulator_Observers\Results_fz4_sensor\k_SA_C_CorridorPod3.mat  SaveFormat=Timeseries  SampleTime=Ts
  nC_bar : Constant  Value=building.zone(12).nC_bar+0.5  SampleTime=Ts
  nH_bar : Constant  Value=building.zone(4).nH_bar  SampleTime=Ts
  nSA_bar : Constant  Value=building.zone(12).nC_bar  SampleTime=Ts
  nZ_bar : Constant  Value=building.zone(12).n_bar  SampleTime=Ts
  xSA_C : ToFile  Filename=C:\Users\papad\Dropbox\Complete_building_simulator_Observers\Results_fz4_sensor\xSA_C_CorridorPod3.mat  SaveFormat=Timeseries  SampleTime=Ts
  Out1 : Outport
  wires:
  Mux3/1 -> Dot Product1/1
  SAGainsThreshold/1 -> Dot Product1/2
  nC_bar/1 -> Mux3/1
  If1/1 -> If Action Subsystem2/Action
  If1/2 -> If Action Subsystem3/Action
  If Action Subsystem2/1(Out1) -> Merge/1  "k=0"
  If Action Subsystem3/1(Out1) -> Merge/2  "k>0"
  Sum3/1 -> If Action Subsystem2/1(In1)
  Sum4/1 -> If Action Subsystem3/1(In1)
  Gain/1 -> Sum4/1, ae_SA_C/1
  Fcn2/1 -> Add2/2, Gain/1  "a^k"
  Dot Product1/1 -> Product5/2, xSA_C/1  "x"
  Product5/1 -> Sum4/3
  Merge/1 -> Out1/1
  a_SA_C/1 -> Mux2/2, Add2/1, Add3/1, Product1/2, Product1/1  "a"
  Mux2/1 -> Fcn2/1
  nSA_bar/1 -> Mux3/4, Sum4/2, Sum3/2  "noise"
  Product1/1 -> Add3/2  "a^2"
  Add2/1 -> Divide/1
  Add3/1 -> Divide/2
  Divide/1 -> aeSA_C_sum/1, Product5/1  "sum"
  ae_SAC/1 -> Sum3/1
  counter/1(Out1) -> k_SA_C/1, If1/1  "k"
  counter/2(Out2) -> Mux2/1  "k>1"
  nH_bar/1 -> Mux3/2
  nZ_bar/1 -> Mux3/3

== system controller_observer_AllAgents_v5/Controller/CorridorPod3_Agent/Threshold_zone ==
  3 : Constant  SampleTime=Ts
  4 : Constant  Value=[ones(numel(building.zone(12).neighbour),1)]  SampleTime=Ts
  Add2 : Sum  Inputs=+-
  Add3 : Sum  Inputs=+-
  Divide : Product  Inputs=*/
  Dot Product1 : DotProduct  SampleTime=Ts  InputSameDT=on  OutDataTypeStr=Inherit: Inherit via internal rule
  Fcn2 : Fcn  Expr=u(2)^(u(1))  SampleTime=Ts
  Gain : Gain  Gain=building.zone(12).e_bar  SampleTime=Ts
  If Action Subsystem2 : Subsystem  TreatAsAtomicUnit=on
  If Action Subsystem3 : Subsystem  TreatAsAtomicUnit=on
  If1 : If  IfExpression=u1 == 0  SampleTime=Ts
  Merge : Merge  InitialOutput=[building.zone(4).e_bar+building.zone(4).n_bar]
  Mux2 : Mux  Inputs=2
  Mux3 : Mux  Inputs=[1 numel(building.zone(12).neighbour) 1 1]
  Product1 : Product  SampleTime=Ts
  Product5 : Product  SampleTime=Ts
  Sum3 : Sum  Inputs=|++  SampleTime=Ts
  Sum4 : Sum  Inputs=+++  SampleTime=Ts
  ZoneGainsThreshold1 : Constant  Value=[building.zone(12).B_z; transpose(building.zone(12).B_z*building.zone(12).n_nei.*building.zone(12).Kij); building.zone(12).B_z*building.zone(12).L; building.zone(12).B_z*building.zone(12).Qbar]  SampleTime=Ts  FramePeriod=Ts
  a mat : ToFile  Filename=C:\Users\papad\Dropbox\Complete_building_simulator_Observers\Results_fz4_sensor\aH_CorridorPod3.mat  SaveFormat=Timeseries  SampleTime=Ts
  a1 : Constant  Value=building.zone(12).a  SampleTime=Ts
  ae1 : Constant  Value=building.zone(12).a*building.zone(12).e_bar  SampleTime=Ts
  ae_sum1 : ToFile  Filename=C:\Users\papad\Dropbox\Complete_building_simulator_Observers\Results_fz4_sensor\aeH_sum_CorridorPod3.mat  SaveFormat=Timeseries  SampleTime=Ts
  ae_z2 : ToFile  Filename=C:\Users\papad\Dropbox\Complete_building_simulator_Observers\Results_fz4_sensor\aeH_CorridorPod3.mat  SaveFormat=Timeseries  SampleTime=Ts
  ae_z3 : ToFile  Filename=C:\Users\papad\Dropbox\Complete_building_simulator_Observers\Results_fz4_sensor\kH_CorridorPod3.mat  SaveFormat=Timeseries  SampleTime=Ts
  counter : Subsystem
  n_bar1 : Constant  Value=building.zone(12).n_bar  SampleTime=Ts
  n_bar2 : Constant  Value=building.zone(4).n_bar  SampleTime=Ts
  x1 : ToFile  Filename=C:\Users\papad\Dropbox\Complete_building_simulator_Observers\Results_fz4_sensor\xH_CorridorPod3.mat  SaveFormat=Timeseries  SampleTime=Ts
  Out1 : Outport
  wires:
  Mux3/1 -> Dot Product1/1
  ZoneGainsThreshold1/1 -> Dot Product1/2
  n_bar1/1 -> Mux3/1
  4/1 -> Mux3/2
  3/1 -> Mux3/4
  If1/1 -> If Action Subsystem2/Action
  If1/2 -> If Action Subsystem3/Action
  If Action Subsystem2/1(Out1) -> Merge/1  "k=0"
  If Action Subsystem3/1(Out1) -> Merge/2  "k>0"
  Sum3/1 -> If Action Subsystem2/1(In1)
  Sum4/1 -> If Action Subsystem3/1(In1)
  Gain/1 -> Sum4/1, ae_z2/1
  Fcn2/1 -> Add2/2, Gain/1  "a^k"
  Dot Product1/1 -> Product5/2, x1/1  "x"
  Product5/1 -> Sum4/3
  Merge/1 -> Out1/1
  a1/1 -> Mux2/2, Add2/1, Add3/1, Product1/2, Product1/1, a mat/1  "a"
  Mux2/1 -> Fcn2/1
  n_bar2/1 -> Sum4/2, Sum3/2, Mux3/3  "noise"
  Product1/1 -> Add3/2  "a^2"
  Add2/1 -> Divide/1
  Add3/1 -> Divide/2
  Divide/1 -> ae_sum1/1, Product5/1  "sum"
  ae1/1 -> Sum3/1
  counter/1(Out1) -> ae_z3/1, If1/1  "k"
  counter/2(Out2) -> Mux2/1  "k>1"

== system controller_observer_AllAgents_v5/Controller/CorridorPod3_Cooling/If Action Subsystem ==
  In1 : Inport
  Action Port : ActionPort  ActionType=then
  Out1 : Outport
  wires:
  In1/1 -> Out1/1

== system controller_observer_AllAgents_v5/Controller/CorridorPod3_Cooling/If Action Subsystem1 ==
  In1 : Inport
  Action Port : ActionPort  ActionType=else
  Out1 : Outport
  wires:
  In1/1 -> Out1/1

== system controller_observer_AllAgents_v5/Controller/CorridorPod3_Heating/If Action Subsystem ==
  In1 : Inport
  Action Port : ActionPort  ActionType=then
  Out1 : Outport
  wires:
  In1/1 -> Out1/1

== system controller_observer_AllAgents_v5/Controller/CorridorPod3_Heating/If Action Subsystem1 ==
  In1 : Inport
  Action Port : ActionPort  ActionType=else
  Out1 : Outport
  wires:
  In1/1 -> Out1/1

== system controller_observer_AllAgents_v5/Controller/EnableDifonifmode1/If Action Subsystem7 ==
  In1 : Inport
  Action Port : ActionPort  InitializeStates=reset  ActionType=then
  Out1 : Outport
  wires:
  In1/1 -> Out1/1

== system controller_observer_AllAgents_v5/Controller/EnableDifonifmode1/If Action Subsystem8 ==
  In1 : Inport
  Action Port : ActionPort  InitializeStates=reset  ActionType=else
  Out1 : Outport
  wires:
  In1/1 -> Out1/1

== system controller_observer_AllAgents_v5/Controller/EnableDifonifmode10/If Action Subsystem7 ==
  In1 : Inport
  Action Port : ActionPort  InitializeStates=reset  ActionType=then
  Out1 : Outport
  wires:
  In1/1 -> Out1/1

== system controller_observer_AllAgents_v5/Controller/EnableDifonifmode10/If Action Subsystem8 ==
  In1 : Inport
  Action Port : ActionPort  InitializeStates=reset  ActionType=else
  Out1 : Outport
  wires:
  In1/1 -> Out1/1

== system controller_observer_AllAgents_v5/Controller/EnableDifonifmode11/If Action Subsystem7 ==
  In1 : Inport
  Action Port : ActionPort  InitializeStates=reset  ActionType=then
  Out1 : Outport
  wires:
  In1/1 -> Out1/1

== system controller_observer_AllAgents_v5/Controller/EnableDifonifmode11/If Action Subsystem8 ==
  In1 : Inport
  Action Port : ActionPort  InitializeStates=reset  ActionType=else
  Out1 : Outport
  wires:
  In1/1 -> Out1/1

== system controller_observer_AllAgents_v5/Controller/EnableDifonifmode12/If Action Subsystem7 ==
  In1 : Inport
  Action Port : ActionPort  InitializeStates=reset  ActionType=then
  Out1 : Outport
  wires:
  In1/1 -> Out1/1

== system controller_observer_AllAgents_v5/Controller/EnableDifonifmode12/If Action Subsystem8 ==
  In1 : Inport
  Action Port : ActionPort  InitializeStates=reset  ActionType=else
  Out1 : Outport
  wires:
  In1/1 -> Out1/1

== system controller_observer_AllAgents_v5/Controller/EnableDifonifmode13/If Action Subsystem7 ==
  In1 : Inport
  Action Port : ActionPort  InitializeStates=reset  ActionType=then
  Out1 : Outport
  wires:
  In1/1 -> Out1/1

== system controller_observer_AllAgents_v5/Controller/EnableDifonifmode13/If Action Subsystem8 ==
  In1 : Inport
  Action Port : ActionPort  InitializeStates=reset  ActionType=else
  Out1 : Outport
  wires:
  In1/1 -> Out1/1

== system controller_observer_AllAgents_v5/Controller/EnableDifonifmode14/If Action Subsystem7 ==
  In1 : Inport
  Action Port : ActionPort  InitializeStates=reset  ActionType=then
  Out1 : Outport
  wires:
  In1/1 -> Out1/1

== system controller_observer_AllAgents_v5/Controller/EnableDifonifmode14/If Action Subsystem8 ==
  In1 : Inport
  Action Port : ActionPort  InitializeStates=reset  ActionType=else
  Out1 : Outport
  wires:
  In1/1 -> Out1/1

== system controller_observer_AllAgents_v5/Controller/EnableDifonifmode15/If Action Subsystem7 ==
  In1 : Inport
  Action Port : ActionPort  InitializeStates=reset  ActionType=then
  Out1 : Outport
  wires:
  In1/1 -> Out1/1

== system controller_observer_AllAgents_v5/Controller/EnableDifonifmode15/If Action Subsystem8 ==
  In1 : Inport
  Action Port : ActionPort  InitializeStates=reset  ActionType=else
  Out1 : Outport
  wires:
  In1/1 -> Out1/1

== system controller_observer_AllAgents_v5/Controller/EnableDifonifmode16/If Action Subsystem7 ==
  In1 : Inport
  Action Port : ActionPort  InitializeStates=reset  ActionType=then
  Out1 : Outport
  wires:
  In1/1 -> Out1/1

== system controller_observer_AllAgents_v5/Controller/EnableDifonifmode16/If Action Subsystem8 ==
  In1 : Inport
  Action Port : ActionPort  InitializeStates=reset  ActionType=else
  Out1 : Outport
  wires:
  In1/1 -> Out1/1

== system controller_observer_AllAgents_v5/Controller/EnableDifonifmode17/If Action Subsystem7 ==
  In1 : Inport
  Action Port : ActionPort  InitializeStates=reset  ActionType=then
  Out1 : Outport
  wires:
  In1/1 -> Out1/1

== system controller_observer_AllAgents_v5/Controller/EnableDifonifmode17/If Action Subsystem8 ==
  In1 : Inport
  Action Port : ActionPort  InitializeStates=reset  ActionType=else
  Out1 : Outport
  wires:
  In1/1 -> Out1/1

== system controller_observer_AllAgents_v5/Controller/EnableDifonifmode18/If Action Subsystem7 ==
  In1 : Inport
  Action Port : ActionPort  InitializeStates=reset  ActionType=then
  Out1 : Outport
  wires:
  In1/1 -> Out1/1

== system controller_observer_AllAgents_v5/Controller/EnableDifonifmode18/If Action Subsystem8 ==
  In1 : Inport
  Action Port : ActionPort  InitializeStates=reset  ActionType=else
  Out1 : Outport
  wires:
  In1/1 -> Out1/1

== system controller_observer_AllAgents_v5/Controller/EnableDifonifmode19/If Action Subsystem7 ==
  In1 : Inport
  Action Port : ActionPort  InitializeStates=reset  ActionType=then
  Out1 : Outport
  wires:
  In1/1 -> Out1/1

== system controller_observer_AllAgents_v5/Controller/EnableDifonifmode19/If Action Subsystem8 ==
  In1 : Inport
  Action Port : ActionPort  InitializeStates=reset  ActionType=else
  Out1 : Outport
  wires:
  In1/1 -> Out1/1

== system controller_observer_AllAgents_v5/Controller/EnableDifonifmode2/If Action Subsystem7 ==
  In1 : Inport
  Action Port : ActionPort  InitializeStates=reset  ActionType=then
  Out1 : Outport
  wires:
  In1/1 -> Out1/1

== system controller_observer_AllAgents_v5/Controller/EnableDifonifmode2/If Action Subsystem8 ==
  In1 : Inport
  Action Port : ActionPort  InitializeStates=reset  ActionType=else
  Out1 : Outport
  wires:
  In1/1 -> Out1/1

== system controller_observer_AllAgents_v5/Controller/EnableDifonifmode20/If Action Subsystem7 ==
  In1 : Inport
  Action Port : ActionPort  InitializeStates=reset  ActionType=then
  Out1 : Outport
  wires:
  In1/1 -> Out1/1

== system controller_observer_AllAgents_v5/Controller/EnableDifonifmode20/If Action Subsystem8 ==
  In1 : Inport
  Action Port : ActionPort  InitializeStates=reset  ActionType=else
  Out1 : Outport
  wires:
  In1/1 -> Out1/1

== system controller_observer_AllAgents_v5/Controller/EnableDifonifmode21/If Action Subsystem7 ==
  In1 : Inport
  Action Port : ActionPort  InitializeStates=reset  ActionType=then
  Out1 : Outport
  wires:
  In1/1 -> Out1/1

== system controller_observer_AllAgents_v5/Controller/EnableDifonifmode21/If Action Subsystem8 ==
  In1 : Inport
  Action Port : ActionPort  InitializeStates=reset  ActionType=else
  Out1 : Outport
  wires:
  In1/1 -> Out1/1

== system controller_observer_AllAgents_v5/Controller/EnableDifonifmode22/If Action Subsystem7 ==
  In1 : Inport
  Action Port : ActionPort  InitializeStates=reset  ActionType=then
  Out1 : Outport
  wires:
  In1/1 -> Out1/1

== system controller_observer_AllAgents_v5/Controller/EnableDifonifmode22/If Action Subsystem8 ==
  In1 : Inport
  Action Port : ActionPort  InitializeStates=reset  ActionType=else
  Out1 : Outport
  wires:
  In1/1 -> Out1/1

== system controller_observer_AllAgents_v5/Controller/EnableDifonifmode23/If Action Subsystem7 ==
  In1 : Inport
  Action Port : ActionPort  InitializeStates=reset  ActionType=then
  Out1 : Outport
  wires:
  In1/1 -> Out1/1

== system controller_observer_AllAgents_v5/Controller/EnableDifonifmode23/If Action Subsystem8 ==
  In1 : Inport
  Action Port : ActionPort  InitializeStates=reset  ActionType=else
  Out1 : Outport
  wires:
  In1/1 -> Out1/1

== system controller_observer_AllAgents_v5/Controller/EnableDifonifmode24/If Action Subsystem7 ==
  In1 : Inport
  Action Port : ActionPort  InitializeStates=reset  ActionType=then
  Out1 : Outport
  wires:
  In1/1 -> Out1/1

== system controller_observer_AllAgents_v5/Controller/EnableDifonifmode24/If Action Subsystem8 ==
  In1 : Inport
  Action Port : ActionPort  InitializeStates=reset  ActionType=else
  Out1 : Outport
  wires:
  In1/1 -> Out1/1

== system controller_observer_AllAgents_v5/Controller/EnableDifonifmode25/If Action Subsystem7 ==
  In1 : Inport
  Action Port : ActionPort  InitializeStates=reset  ActionType=then
  Out1 : Outport
  wires:
  In1/1 -> Out1/1

== system controller_observer_AllAgents_v5/Controller/EnableDifonifmode25/If Action Subsystem8 ==
  In1 : Inport
  Action Port : ActionPort  InitializeStates=reset  ActionType=else
  Out1 : Outport
  wires:
  In1/1 -> Out1/1

== system controller_observer_AllAgents_v5/Controller/EnableDifonifmode26/If Action Subsystem7 ==
  In1 : Inport
  Action Port : ActionPort  InitializeStates=reset  ActionType=then
  Out1 : Outport
  wires:
  In1/1 -> Out1/1

== system controller_observer_AllAgents_v5/Controller/EnableDifonifmode26/If Action Subsystem8 ==
  In1 : Inport
  Action Port : ActionPort  InitializeStates=reset  ActionType=else
  Out1 : Outport
  wires:
  In1/1 -> Out1/1

== system controller_observer_AllAgents_v5/Controller/EnableDifonifmode27/If Action Subsystem7 ==
  In1 : Inport
  Action Port : ActionPort  InitializeStates=reset  ActionType=then
  Out1 : Outport
  wires:
  In1/1 -> Out1/1

== system controller_observer_AllAgents_v5/Controller/EnableDifonifmode27/If Action Subsystem8 ==
  In1 : Inport
  Action Port : ActionPort  InitializeStates=reset  ActionType=else
  Out1 : Outport
  wires:
  In1/1 -> Out1/1

== system controller_observer_AllAgents_v5/Controller/EnableDifonifmode28/If Action Subsystem7 ==
  In1 : Inport
  Action Port : ActionPort  InitializeStates=reset  ActionType=then
  Out1 : Outport
  wires:
  In1/1 -> Out1/1

== system controller_observer_AllAgents_v5/Controller/EnableDifonifmode28/If Action Subsystem8 ==
  In1 : Inport
  Action Port : ActionPort  InitializeStates=reset  ActionType=else
  Out1 : Outport
  wires:
  In1/1 -> Out1/1

== system controller_observer_AllAgents_v5/Controller/EnableDifonifmode29/If Action Subsystem7 ==
  In1 : Inport
  Action Port : ActionPort  InitializeStates=reset  ActionType=then
  Out1 : Outport
  wires:
  In1/1 -> Out1/1

== system controller_observer_AllAgents_v5/Controller/EnableDifonifmode29/If Action Subsystem8 ==
  In1 : Inport
  Action Port : ActionPort  InitializeStates=reset  ActionType=else
  Out1 : Outport
  wires:
  In1/1 -> Out1/1

== system controller_observer_AllAgents_v5/Controller/EnableDifonifmode3/If Action Subsystem7 ==
  In1 : Inport
  Action Port : ActionPort  InitializeStates=reset  ActionType=then
  Out1 : Outport
  wires:
  In1/1 -> Out1/1

== system controller_observer_AllAgents_v5/Controller/EnableDifonifmode3/If Action Subsystem8 ==
  In1 : Inport
  Action Port : ActionPort  InitializeStates=reset  ActionType=else
  Out1 : Outport
  wires:
  In1/1 -> Out1/1

== system controller_observer_AllAgents_v5/Controller/EnableDifonifmode30/If Action Subsystem7 ==
  In1 : Inport
  Action Port : ActionPort  InitializeStates=reset  ActionType=then
  Out1 : Outport
  wires:
  In1/1 -> Out1/1

== system controller_observer_AllAgents_v5/Controller/EnableDifonifmode30/If Action Subsystem8 ==
  In1 : Inport
  Action Port : ActionPort  InitializeStates=reset  ActionType=else
  Out1 : Outport
  wires:
  In1/1 -> Out1/1

== system controller_observer_AllAgents_v5/Controller/EnableDifonifmode31/If Action Subsystem7 ==
  In1 : Inport
  Action Port : ActionPort  InitializeStates=reset  ActionType=then
  Out1 : Outport
  wires:
  In1/1 -> Out1/1

== system controller_observer_AllAgents_v5/Controller/EnableDifonifmode31/If Action Subsystem8 ==
  In1 : Inport
  Action Port : ActionPort  InitializeStates=reset  ActionType=else
  Out1 : Outport
  wires:
  In1/1 -> Out1/1

== system controller_observer_AllAgents_v5/Controller/EnableDifonifmode32/If Action Subsystem7 ==
  In1 : Inport
  Action Port : ActionPort  InitializeStates=reset  ActionType=then
  Out1 : Outport
  wires:
  In1/1 -> Out1/1

== system controller_observer_AllAgents_v5/Controller/EnableDifonifmode32/If Action Subsystem8 ==
  In1 : Inport
  Action Port : ActionPort  InitializeStates=reset  ActionType=else
  Out1 : Outport
  wires:
  In1/1 -> Out1/1

== system controller_observer_AllAgents_v5/Controller/EnableDifonifmode33/If Action Subsystem7 ==
  In1 : Inport
  Action Port : ActionPort  InitializeStates=reset  ActionType=then
  Out1 : Outport
  wires:
  In1/1 -> Out1/1

== system controller_observer_AllAgents_v5/Controller/EnableDifonifmode33/If Action Subsystem8 ==
  In1 : Inport
  Action Port : ActionPort  InitializeStates=reset  ActionType=else
  Out1 : Outport
  wires:
  In1/1 -> Out1/1

== system controller_observer_AllAgents_v5/Controller/EnableDifonifmode34/If Action Subsystem7 ==
  In1 : Inport
  Action Port : ActionPort  InitializeStates=reset  ActionType=then
  Out1 : Outport
  wires:
  In1/1 -> Out1/1

== system controller_observer_AllAgents_v5/Controller/EnableDifonifmode34/If Action Subsystem8 ==
  In1 : Inport
  Action Port : ActionPort  InitializeStates=reset  ActionType=else
  Out1 : Outport
  wires:
  In1/1 -> Out1/1
